simulink library "Renault_Library"  (Simulink 9.2)
settings: Solver=ode3  StartTime=0.0  StopTime=10.0  FixedStep=0.020  RelTol=1e-3  EnableMultiTasking=on
undefined symbols (not defined in the file or in its folder): ABC, AssystemProposal, b, chkval, CoeffA_C, CoeffB_C, CoeffMean_C, ConfTime_C, DiagDelay_C, DividePercent, FailureConfActive_C, FallingEdgeDuration_C, HighLevelThresholdVoltage_C, HighLevelVoltage_C, HighLevelVoltageTunable_C, InitValue_C, InputVoltRangeMax_C, InputVoltRangeMin_C, LowActiveContact_C, LowLevelThresholdVoltage_C, LowLevelVoltage_C, MaxOperatingVoltage_C, MinOperatingVoltage_C, mV2V_conversion, OUT1, OutOfRangeHigh_C, OutOfRangeLow_C, PinSupplySaturationMax, PinSupplySaturationMin, Res1Value_C, Res2Value_C, Res3Value_C, RisingEdgeDuration_C, RSA_CANSignalFailMonitoring, SampleTime_C, service14Table, service19Table, service22Table, service24Table, service28Table, service2ETable, service2FTable, service31Table, service87Table, SizeXStateDID2FTable, SizeXTable14, SizeXTable19, SizeXTable22, SizeXTable24, SizeXTable28, SizeXTable2E, SizeXTable2F, SizeXTable31, SizeXTable87, SizeYStateDID2FTable, SizeYTable14, SizeYTable19, SizeYTable22, SizeYTable24, SizeYTable28 ... (+22)
undefined symbols (not defined in the file): BatteryVoltage_ActivationThreshold_C, FrameStatus_AbsentValue_C, FrameStatus_ConfAbsentValue_C, MemThreshold_C, NAMEOFTHEMODEL_SAMPLE_TIME_CST, NbAbs1_C, NbConfAbs1_C, NbConfPres1_C, NbPres1_C, NOMDUSIGNAL_FaultConfTime_C, NOMDUSIGNAL_FaultRehabTime_C, NOMDUSIGNAL_switchvalue_C, NOMDUSIGNAL_unavailablevalue_C, PeriodFrame1_C, ResetTime_C, WakeUpTime_C
libraries: $bdroot x112, simulink x75
(part 8 of 32)

== system Renault_Library/Standard blocks/Logic & Bit Operations ==
  note: /!\ DO NOT USE THIS RED BLOCKS ANYMORE /!\
  note: Logic And Bit Operation
  Bit_Clear1 : simulink/Logic and Bit Operations/Bit Clear  SystemSampleTime=-1  iBit=0
      settings: SourceProductName=Simulink  SourceProductBaseCode=SL
  Bit_Set : simulink/Logic and Bit Operations/Bit Set  SystemSampleTime=-1  iBit=0
      settings: SourceProductName=Simulink  SourceProductBaseCode=SL
  BitwiseOperator : S-Function  FunctionName=sfix_bitop  Parameters=UseBitMask,NumInputPorts,BitMask,BitMaskRealWorld,logicop  SFunctionDeploymentMode=off  EnableBusSupport=off  mask(Bitwise Operator){logicop=AND, UseBitMask=on, NumInputPorts=1, BitMask=bin2dec('11011001'), BitMaskRealWorld=Stored Integer}
      mask prompts: logicop "Operator:"; UseBitMask "Use bit mask ..."; NumInputPorts "Number of input ports:"; BitMask "Bit Mask"; BitMaskRealWorld "Treat mask as:"
      mask help: Perform the specified bitwise operation on the inputs. The output data type should represent zero exactly.
      mask init:
      | str = '';
      | if UseBitMask
      |   [m,n] = size(BitMask);
      |   if ischar(BitMask)
      |     len = m;
      |     n = 1;
      |   else
      |     len = m * n;
      |   end
      |   internalBitMask = zeros(m,n);
      |   if isnumeric(BitMask)
      |     for i=1:len,
      |       internalBitMask(i) = BitMask(i);
      |     end
      |   elseif ischar(BitMask)
      |     for i=1:m,
      |       internalBitMask(i) = hex2dec(BitMask(i,:));
      |     end
      |   elseif iscell(BitMask)
      |     for i=1:len,
      |       if isnumeric( BitMask{i} )
      |         internalBitMask(i) = BitMask{i};
      |       elseif ischar( BitMask{i} )
      |         internalBitMask(i) = hex2dec(BitMask{i});
      |       else
      |         errordlg(['Data type is not supported at cell item ', num2str(i) ]);
      |       end
      |     end
      |   end
      |   BitMask = internalBitMask;
      |   for i=1:len
      |     if BitMask(i) > (2^52)-1
      |       error(DAStudio.message('Simulink:blocks:BitMaskWordLengthTooLarge')); 
      |     end
      |   end 
      |   if len == 1
      |      if BitMaskRealWorld == 1 || BitMask(1) < 0
      |        str=num2str(BitMask(1));
      |      else
      |        str=['0x',dec2hex(floor(BitMask(1)))];
      |      end
      |   else
      |      str='Masks';
      |   end
      | else
      |   BitMask = 0;
      |   str='';
      | end
      mask TypeOptions logicop: AND | OR | NAND | NOR | XOR | NOT
      mask Callback logicop: fixptdialog(gcbh)
      mask Callback UseBitMask: fixptdialog(gcbh)
      mask TypeOptions BitMaskRealWorld: Real World Value | Stored Integer
      mask Display: fprintf(['Bitwise\n',logicop,'\n',str])
  Bitwise_Operator : simulink/Logic and Bit Operations/Bitwise Operator  logicop=AND  UseBitMask=on  BitMask=bin2dec('11011001')  BitMaskRealWorld=Stored Integer
  Extract Bits : Subsystem  mask(Extract Bits){bitsToExtract=Upper half, numBits=8, bitIdxRange=[0 7], outScalingMode=Preserve fixed-point scaling}
      mask prompts: bitsToExtract "Bits to extract:"; numBits "Number of bits:"; bitIdxRange "Bit indices ([start end], 0-based relative to LSB):"; outScalingMode "Output scaling mode:"
      mask help: Output selected bits from each of the fixed-point input samples. Selecting "Upper half" or "Lower half" results in a positive number of bits in the output word length, according to the equation numOutputBits = ceil(numInputBits / 2).
      mask init:
      | str = fixpt_extractbits_mask('icon', numBits, bitIdxRange); 
      | fixpt_extractbits_mask_script;
      mask TypeOptions bitsToExtract: Upper half | Lower half | Range starting with most significant bit | Range ending with least significant bit | Range of bits
      mask Callback bitsToExtract: fixpt_extractbits_mask;
      mask TypeOptions outScalingMode: Preserve fixed-point scaling | Treat bit field as an integer
      mask Display: disp(str);
  Extract_Bits : simulink/Logic and Bit Operations/Extract Bits  SystemSampleTime=-1  bitsToExtract=Upper half  numBits=8  bitIdxRange=[0 7]  outScalingMode=Preserve fixed-point scaling
      settings: SourceProductName=Simulink
  LogicalOperator : Logic  OutDataTypeStr=boolean
  RelationalOperator : RelationalOperator  Operator=<=  OutDataTypeStr=boolean  RndMeth=Nearest  InputSameDT=on
  ShiftArithmetic : ArithShift  BitShiftNumber=8

== system Renault_Library/Standard blocks/Math Operations ==
  note: Math Operations
  Abs : Abs
  Add : Sum  OutDataTypeStr=Inherit: Inherit via back propagation  RndMeth=Zero  InputSameDT=on
      settings: AttributesFormatString=SAT = %<SaturateOnIntegerOverflow>
  Assignment : Assignment  IndexOptions=Index vector (dialog)  Indices=1  OutputSizes=1
  Divide : Product  Inputs=*/  RndMeth=Zero
  MinMax : MinMax  RndMeth=Zero  InputSameDT=on
  Product : Product  RndMeth=Zero
  Sign : Signum
  SignedSqrt : Sqrt  Operator=signedSqrt  SaturateOnIntegerOverflow=on
  Sqrt : Sqrt  SaturateOnIntegerOverflow=on
  Subtract : Sum  Inputs=+-  OutDataTypeStr=Inherit: Inherit via back propagation  RndMeth=Zero  InputSameDT=on
      settings: AttributesFormatString=SAT = %<SaturateOnIntegerOverflow>
  Sum : Sum  Inputs=|++  OutDataTypeStr=Inherit: Inherit via back propagation  RndMeth=Zero  InputSameDT=on
      settings: AttributesFormatString=SAT = %<SaturateOnIntegerOverflow>
  SumofElements : Sum  Inputs=+  OutDataTypeStr=Inherit: Inherit via back propagation  RndMeth=Zero  InputSameDT=on
      settings: AttributesFormatString=SAT = %<SaturateOnIntegerOverflow>

== system Renault_Library/Standard blocks/Model Wide Utilities ==
  note: /!\ DO NOT USE THIS RED BLOCKS ANYMORE /!\
  note: Model Wide Utilities
  DocBlock : Subsystem  mask(DocBlock){ECoderFlag=, DocumentType=Text}
      mask prompts: ECoderFlag "Simulink:blocks:docblockEmbeddedCoderFlagLabel"; DocumentType "Simulink:blocks:docblockDocTypeLabel"
      mask help: Use this block to save long descriptive text with the model. Double-clicking the block will open an editor.
      settings: DeleteFcn=docblock('close_document',gcb);  PreSaveFcn=docblock('save_document',gcb);  OpenFcn=docblock('edit_document',gcb);
      mask TypeOptions DocumentType: Text | RTF | HTML
      mask Display:
      | plot([.8 0 0 1 1 .8 .8 1],[1 1 0 0 .8 1 .8 .8]);
      | text(.5,.6,'DOC','horizontalalignment','center');
      | text(.95,.05,get_param(gcb,'DocumentType'),'verticalalignment','bottom','horizontalalignment','right');
  ModelInfo : Subsystem  LoadFcn=slcm LoadBlock;  InitFcn=slcm('UpdateAllCMBlocksInThisModelInit')  mask(CMBlock){InitialSaveTempField=, InitialBlockCM=None, BlockCM=none, Frame=on, SaveTempField=, DisplayStringWithTags=Model Info, MaskDisplayString=Model Info, HorizontalTextAlignment=Center, LeftAlignmentValue=0.5, SourceBlockDiagram=Renault_Library, TagMaxNumber=20, CMTag1=, CMTag2=, CMTag3=, CMTag4=, CMTag5=, CMTag6=, CMTag7=, CMTag8=, CMTag9=, CMTag10=, CMTag11=, CMTag12=, CMTag13=, CMTag14=, CMTag15=, CMTag16=, CMTag17=, CMTag18=, CMTag19=, CMTag20=}
      mask help: This block allows revision control information to be displayed within the model.
      settings: CopyFcn=slcm CopyBlock;  DeleteFcn=slcm Cancel;  PostSaveFcn=slcm('UpdateAllCMBlocksInThisModel')  NameChangeFcn=slcm Rename;  OpenFcn=slcm
      mask Display: text(str2num(LeftAlignmentValue), 0.5, MaskDisplayString, 'HorizontalAlignment', HorizontalTextAlignment)
  Model_Info : simulink/Model-Wide Utilities/Model Info  SystemSampleTime=-1  InitialBlockCM=none  BlockCM=none  Frame=on  DisplayStringWithTags=Model Info  HorizontalTextAlignment=Center  LeftAlignmentValue=0.5  SourceBlockDiagram=Renault_Library_11  TagMaxNumber=20

== system Renault_Library/Standard blocks/Ports & Subsystems ==
  note: Ports and Subsystems
  In : Inport
  Enable : EnablePort
      settings: AttributesFormatString=State : %<StatesWhenEnabling>
  Trigger : TriggerPort  VariantControl=(inherit)
      settings: AttributesFormatString=State : %<StatesWhenEnabling>
  AtomicSubsystem : Subsystem  TreatAsAtomicUnit=on  mask(Atomic Subsystem){}
      mask help: A subsystem block template containing an inport and outport block.
      settings: CopyFcn=set_param(gcbh,'LinkStatus','none'); set_param(gcbh,'Mask','off'); set_param(gcbh,'MaskType',''); set_param(gcbh,'MaskDescription',''); set_param(gcbh,'OpenFcn',''); set_param(gcbh,'CopyFcn','')  OpenFcn=open_system(gcbh,'force')
  CodeReuseSubsystem : Subsystem  TreatAsAtomicUnit=on  mask(Code Reuse Subsystem){}
      mask help: A subsystem block template containing an inport and outport block.
      settings: CopyFcn=set_param(gcbh,'LinkStatus','none'); set_param(gcbh,'Mask','off'); set_param(gcbh,'MaskType',''); set_param(gcbh,'MaskDescription',''); set_param(gcbh,'OpenFcn',''); set_param(gcbh,'CopyFcn','')  OpenFcn=open_system(gcbh,'force')  RTWFcnNameOpts=Use subsystem name  RTWFileNameOpts=Use function name
  EnabledSubsystem : Subsystem  TreatAsAtomicUnit=on  mask(Enabled Subsystem){}
      mask help: A subsystem block template containing an enable port, inport and outport block.
      settings: CopyFcn=set_param(gcbh,'LinkStatus','none'); set_param(gcbh,'Mask','off'); set_param(gcbh,'MaskType',''); set_param(gcbh,'MaskDescription',''); set_param(gcbh,'OpenFcn',''); set_param(gcbh,'CopyFcn','')  OpenFcn=open_system(gcbh,'force')
  EnabledandTriggeredSubsystem : Subsystem  TreatAsAtomicUnit=on  mask(Enabled And Triggered Subsystem){}
      mask help: A subsystem block template containing an enable port, trigger port, inport and outport block.
      settings: CopyFcn=set_param(gcbh,'LinkStatus','none'); set_param(gcbh,'Mask','off'); set_param(gcbh,'MaskType',''); set_param(gcbh,'MaskDescription',''); set_param(gcbh,'OpenFcn',''); set_param(gcbh,'CopyFcn','');  OpenFcn=open_system(gcbh,'force');
  ForIteratorSubsystem : Subsystem  TreatAsAtomicUnit=on  mask(For Iterator Subsystem){}
      mask help: A subsystem block template containing a for iterator, inport and outport block.
      settings: CopyFcn=set_param(gcbh,'LinkStatus','none'); set_param(gcbh,'Mask','off'); set_param(gcbh,'MaskType',''); set_param(gcbh,'MaskDescription',''); set_param(gcbh,'OpenFcn',''); set_param(gcbh,'CopyFcn','')  OpenFcn=open_system(gcbh,'force')
  FunctionCallSubsystem : Subsystem  TreatAsAtomicUnit=on  mask(Function-Call Subsystem){}
      mask help: A subsystem block template containing a function-call trigger port, inport and outport block.
      settings: CopyFcn=set_param(gcbh,'LinkStatus','none'); set_param(gcbh,'Mask','off'); set_param(gcbh,'MaskType',''); set_param(gcbh,'MaskDescription',''); set_param(gcbh,'OpenFcn',''); set_param(gcbh,'CopyFcn','');  OpenFcn=open_system(gcbh,'force');
  FunctionCall_Generator : simulink/Ports & Subsystems/Function-Call Generator  sample_time=1  numberOfIterations=1
  If : If
  IfActionSubsystem : Subsystem  TreatAsAtomicUnit=on  mask(If Action Subsystem){}
      mask help: A subsystem block template containing an action port, inport and outport block.
      settings: CopyFcn=set_param(gcbh,'LinkStatus','none'); set_param(gcbh,'Mask','off'); set_param(gcbh,'MaskType',''); set_param(gcbh,'MaskDescription',''); set_param(gcbh,'OpenFcn',''); set_param(gcbh,'CopyFcn','')  OpenFcn=open_system(gcbh,'force')
  Model : ModelReference  SimulationMode=Normal
  Model_Variants : ModelReference  SimulationMode=Normal  Variant=on
      settings: AttributesFormatString=%<ActiveVariant>
  Subsystem : Subsystem  mask(Virtual Subsystem){}
      mask help: A subsystem block template containing an inport and outport block.
      settings: CopyFcn=set_param(gcbh,'LinkStatus','none'); set_param(gcbh,'Mask','off'); set_param(gcbh,'MaskType',''); set_param(gcbh,'MaskDescription',''); set_param(gcbh,'OpenFcn',''); set_param(gcbh,'CopyFcn','')  OpenFcn=open_system(gcbh,'force')
  SwitchCase : SwitchCase
  SwitchCaseActionSubsystem : Subsystem  TreatAsAtomicUnit=on  mask(Switch Case Action Subsystem){}
      mask help: A subsystem block template containing an action port, inport and outport block.
      settings: CopyFcn=set_param(gcbh,'LinkStatus','none'); set_param(gcbh,'Mask','off'); set_param(gcbh,'MaskType',''); set_param(gcbh,'MaskDescription',''); set_param(gcbh,'OpenFcn',''); set_param(gcbh,'CopyFcn','')  OpenFcn=open_system(gcbh,'force')
  SwitchCaseActionSubsystem1 : Subsystem  TreatAsAtomicUnit=on  mask(Switch Case Action Subsystem){}
      mask help: A subsystem block template containing an action port, inport and outport block.
      settings: CopyFcn=set_param(gcbh,'LinkStatus','none'); set_param(gcbh,'Mask','off'); set_param(gcbh,'MaskType',''); set_param(gcbh,'MaskDescription',''); set_param(gcbh,'OpenFcn',''); set_param(gcbh,'CopyFcn','')  OpenFcn=open_system(gcbh,'force')
  TriggeredSubsystem : Subsystem  TreatAsAtomicUnit=on  mask(Triggered Subsystem){}
      mask help: A subsystem block template containing a trigger port, inport and outport block.
      settings: CopyFcn=set_param(gcbh,'LinkStatus','none'); set_param(gcbh,'Mask','off'); set_param(gcbh,'MaskType',''); set_param(gcbh,'MaskDescription',''); set_param(gcbh,'OpenFcn',''); set_param(gcbh,'CopyFcn','')  OpenFcn=open_system(gcbh,'force')
  Variant_Subsystem : Subsystem  Variant=on  mask(Variant Subsystem){}
      mask help: A Variant Subsystem template containing an inport and outport block
      settings: CopyFcn=set_param(gcbh,'LinkStatus','none'); set_param(gcbh,'Mask','off'); set_param(gcbh,'MaskType',''); set_param(gcbh,'MaskDescription',''); set_param(gcbh,'OpenFcn',''); set_param(gcbh,'CopyFcn','')
  WhileIteratorSubsystem : Subsystem  TreatAsAtomicUnit=on  mask(While Iterator Subsystem){}
      mask help: A subsystem block template containing a while iterator, inport and outport block.
      settings: CopyFcn=set_param(gcbh,'LinkStatus','none'); set_param(gcbh,'Mask','off'); set_param(gcbh,'MaskType',''); set_param(gcbh,'MaskDescription',''); set_param(gcbh,'OpenFcn',''); set_param(gcbh,'CopyFcn','')  OpenFcn=open_system(gcbh,'force')
  Out : Outport
  wires:
  SwitchCase/2 -> SwitchCaseActionSubsystem/Action
  SwitchCase/1 -> SwitchCaseActionSubsystem1/Action
  If/1 -> IfActionSubsystem/Action

== system Renault_Library/Standard blocks/Signal Attributes ==
  note: Signal Attibutes
  DataTypeConversion : DataTypeConversion  RndMeth=Zero

== system Renault_Library/Standard blocks/Signal Routing ==
  note: Signal Routing
  BusCreator2 : BusCreator  Inputs=2
  BusSelector2 : BusSelector  OutputSignals=signal1,signal2
  Demux2 : Demux  Outputs=2
  IndexVector : MultiPortSwitch  DataPortOrder=Zero-based contiguous  Inputs=1  zeroidx=on  RndMeth=Zero  InputSameDT=on
      settings: AttributesFormatString=PortOrder=%<DataPortOrder>
  Merge : Merge
  MultiportSwitch : MultiPortSwitch  DataPortOrder=Zero-based contiguous  zeroidx=on  RndMeth=Zero  InputSameDT=on
      settings: AttributesFormatString=PortOrder=%<DataPortOrder>
  Mux2 : Mux  Inputs=2
  Selector : Selector  InputPortWidth=3  IndexOptions=Index vector (dialog)  Indices=[1 3]  OutputSizes=1
  Switch : Switch  Criteria=u2 ~= 0  RndMeth=Zero  InputSameDT=on
      settings: AttributesFormatString=Criteria=%<Criteria>

== system Renault_Library/Standard blocks/Sinks ==
  note: Sink
  Terminator : Terminator
  Out : Outport

== system Renault_Library/Standard blocks/Sources ==
  note: Sources
  In : Inport
  Constant : Constant  Value=ABC
  Enumerated_Constant : simulink/Sources/Enumerated Constant  SystemSampleTime=-1  OutDataTypeStr=Enum: SlDemoSign  Value=SlDemoSign.Positive  SampleTime=-1
      settings: SourceProductName=Simulink

== system Renault_Library/Complex blocks/Discontinuities/RSA_DetectionSaturation ==
  note: 0 : pas saturation
      | 1: saturation min
      |  2: saturation max
      | 3: saturation avec min limite = min limite
  Threshold_Upper : Inport
      settings: AttributesFormatString=Type : %<OutDataTypeStr>
  In : Inport  Port=2
      settings: AttributesFormatString=Type : %<OutDataTypeStr>
  Threshold_Lower : Inport  Port=3
      settings: AttributesFormatString=Type : %<OutDataTypeStr>
  Constant : Constant  Value=SATMIN  OutDataTypeStr=Inherit: Inherit via back propagation  SampleTime=-1
  Constant1 : Constant  Value=SATMAX  OutDataTypeStr=Inherit: Inherit via back propagation  SampleTime=-1
  Constant2 : Constant  Value=SATPAS  OutDataTypeStr=uint8  SampleTime=-1
  Max : MinMax  Function=max  Inputs=2  OutDataTypeStr=Inherit: Inherit via back propagation  RndMeth=Zero  SaturateOnIntegerOverflow=on  InputSameDT=on
  Min : MinMax  Inputs=2  OutDataTypeStr=Inherit: Inherit via back propagation  RndMeth=Zero  SaturateOnIntegerOverflow=on  InputSameDT=on
  RelationalOperator : RelationalOperator  Operator===  OutDataTypeStr=boolean  RndMeth=Nearest  InputSameDT=on
  RelationalOperator1 : RelationalOperator  Operator===  OutDataTypeStr=boolean  RndMeth=Nearest  InputSameDT=on
  Sum : Sum  Inputs=2  OutDataTypeStr=Inherit: Inherit via back propagation  RndMeth=Zero  SaturateOnIntegerOverflow=on  InputSameDT=on
      settings: AttributesFormatString=SAT = %<SaturateOnIntegerOverflow>
  Switch : Switch  Criteria=u2 ~= 0  RndMeth=Zero  InputSameDT=on
      settings: AttributesFormatString=Criteria=%<Criteria>
  Switch1 : Switch  Criteria=u2 ~= 0  RndMeth=Zero  InputSameDT=on
      settings: AttributesFormatString=Criteria=%<Criteria>
  Out : Outport
      settings: AttributesFormatString=Type : %<OutDataTypeStr>\nInit :%<InitialOutput>
  sat : Outport  Port=2
      settings: AttributesFormatString=Type : %<OutDataTypeStr>\nInit :%<InitialOutput>
  wires:
  In/1 -> Max/1
  Threshold_Lower/1 -> RelationalOperator1/2, Max/2
  Max/1 -> Min/1
  Min/1 -> RelationalOperator1/1, RelationalOperator/1, Out/1
  RelationalOperator/1 -> Switch/2
  Sum/1 -> sat/1
  Switch/1 -> Sum/1
  Switch1/1 -> Sum/2
  RelationalOperator1/1 -> Switch1/2
  Constant/1 -> Switch1/1
  Constant1/1 -> Switch/1
  [Nsx_Zero] -> Switch1/3
  [Nsx_Zero] -> Switch/3
  Constant2/1 -> [Nsx_Zero]
  Threshold_Upper/1 -> [Threshold_Upper]
  [Threshold_Upper] -> Min/2
  [Threshold_Upper] -> RelationalOperator/2

== system Renault_Library/Complex blocks/Discontinuities/RSA_Hysteresis ==
  High_Threshold : Inport
      settings: AttributesFormatString=Type : %<OutDataTypeStr>
  In : Inport  Port=2
      settings: AttributesFormatString=Type : %<OutDataTypeStr>
  Low_Threshold : Inport  Port=3
      settings: AttributesFormatString=Type : %<OutDataTypeStr>
  DataTypeConversion : DataTypeConversion  RndMeth=Zero
  FixPt_Relational_Operator : RelationalOperator  OutDataTypeStr=boolean  ZeroCross=off  RndMeth=Nearest  InputSameDT=on
  FixPt_Relational_Operator1 : RelationalOperator  Operator=<=  OutDataTypeStr=boolean  ZeroCross=off  RndMeth=Nearest  InputSameDT=on
  Logical_Operator : Logic  Operator=OR  OutDataTypeStr=boolean
  Logical_Operator1 : Logic  Operator=NOT  OutDataTypeStr=boolean
  Logical_Operator2 : Logic  OutDataTypeStr=boolean
  Unit_Delay : UnitDelay  InitialCondition=HYSINI  SampleTime=-1
  Out : Outport
      settings: AttributesFormatString=Type : %<OutDataTypeStr>\nInit :%<InitialOutput>
  wires:
  DataTypeConversion/1 -> Out/1
  [In] -> FixPt_Relational_Operator/1
  In/1 -> [In]
  [In] -> FixPt_Relational_Operator1/1
  FixPt_Relational_Operator1/1 -> Logical_Operator1/1
  FixPt_Relational_Operator/1 -> Logical_Operator/2
  Low_Threshold/1 -> FixPt_Relational_Operator1/2
  High_Threshold/1 -> FixPt_Relational_Operator/2
  Unit_Delay/1 -> Logical_Operator/1
  Logical_Operator2/1 -> DataTypeConversion/1, Unit_Delay/1  "X3"
  Logical_Operator1/1 -> Logical_Operator2/2  "X2"
  Logical_Operator/1 -> Logical_Operator2/1  "X1"

== system Renault_Library/Complex blocks/Discontinuities/RSA_Hysteresis1 ==
  Input : Inport  Tag=AM,In,0,1,Name,C;CMP,Port,PortWidth,SampleTime;
      settings: AttributesFormatString=Type : %<OutDataTypeStr>
  Threshold1 : Inport  Tag=AM,In,0,1,Name,C;CMP,Port,PortWidth,SampleTime;  Port=2
      settings: AttributesFormatString=Type : %<OutDataTypeStr>
  Threshold2 : Inport  Tag=AM,In,0,1,Name,C;CMP,Port,PortWidth,SampleTime;  Port=3
      settings: AttributesFormatString=Type : %<OutDataTypeStr>
  Value1 : Inport  Tag=AM,In,0,1,Name,C;CMP,Port,PortWidth,SampleTime;  Port=4
      settings: AttributesFormatString=Type : %<OutDataTypeStr>
  Value2 : Inport  Tag=AM,In,0,1,Name,C;CMP,Port,PortWidth,SampleTime;  Port=5
      settings: AttributesFormatString=Type : %<OutDataTypeStr>
  DefaultValue : Inport  Tag=AM,In,0,1,Name,C;CMP,Port,PortWidth,SampleTime;  Port=6
      settings: AttributesFormatString=Type : %<OutDataTypeStr>
  Constant1 : Constant  Value=Th1_Strict  OutDataTypeStr=boolean  SampleTime=-1
  Constant2 : Constant  Value=Th2_Strict  OutDataTypeStr=boolean  SampleTime=-1
  DataTypeConversion : DataTypeConversion  RndMeth=Zero
  RSA_UnitDelayExternalIC : $bdroot/Complex blocks/Discrete/RSA_UnitDelayExternalIC  Tag=[LIB]
  RelationalOperator : RelationalOperator  Tag=AM,Std,2,1;CMP,Operator;  OutDataTypeStr=boolean  RndMeth=Nearest  InputSameDT=on
  RelationalOperator1 : RelationalOperator  Tag=AM,Std,2,1;CMP,Operator;  OutDataTypeStr=boolean  RndMeth=Nearest  InputSameDT=on
  RelationalOperator2 : RelationalOperator  Tag=AM,Std,2,1;CMP,Operator;  Operator=>  OutDataTypeStr=boolean  RndMeth=Nearest  InputSameDT=on
  RelationalOperator3 : RelationalOperator  Tag=AM,Std,2,1;CMP,Operator;  Operator=>  OutDataTypeStr=boolean  RndMeth=Nearest  InputSameDT=on
  Switch : Switch  Tag=GEN,Threshold;AM,Switch,2,1;CMP,Threshold;  Criteria=u2 ~= 0  RndMeth=Zero  SaturateOnIntegerOverflow=on  InputSameDT=on
      settings: AttributesFormatString=Criteria=%<Criteria>
  Switch1 : Switch  Tag=GEN,Threshold;AM,Switch,2,1;CMP,Threshold;  Criteria=u2 ~= 0  RndMeth=Zero  SaturateOnIntegerOverflow=on  InputSameDT=on
      settings: AttributesFormatString=Criteria=%<Criteria>
  Switch2 : Switch  Criteria=u2 ~= 0  RndMeth=Zero  InputSameDT=on
      settings: AttributesFormatString=Criteria=%<Criteria>
  Switch3 : Switch  Criteria=u2 ~= 0  RndMeth=Zero  InputSameDT=on
      settings: AttributesFormatString=Criteria=%<Criteria>
  Output : Outport  Tag=AM,Out,1,0,Name,NC;CMP,Port,OutputWhenDisabled,InitialOutput;
      settings: AttributesFormatString=Type : %<OutDataTypeStr>\nInit :%<InitialOutput>
  wires:
  RSA_UnitDelayExternalIC/1 -> Switch/3
  Switch/1 -> Switch1/3
  Switch1/1 -> RSA_UnitDelayExternalIC/1, DataTypeConversion/1
  Threshold2/1 -> [Threshold2]
  Threshold1/1 -> [Threshold1]
  [Value1] -> Switch1/1
  RelationalOperator1/1 -> Switch2/1
  Constant1/1 -> Switch2/2
  RelationalOperator2/1 -> Switch2/3
  RelationalOperator/1 -> Switch3/1
  Constant2/1 -> Switch3/2
  RelationalOperator3/1 -> Switch3/3
  Switch3/1 -> Switch/2
  Switch2/1 -> Switch1/2
  [Threshold1] -> RelationalOperator1/1
  [Threshold1] -> RelationalOperator2/1
  [Threshold2] -> RelationalOperator/2
  [Threshold2] -> RelationalOperator3/2
  Input/1 -> RelationalOperator1/2, RelationalOperator2/2, RelationalOperator/1, RelationalOperator3/1
  DefaultValue/1 -> [DefaultValue]
  [DefaultValue] -> RSA_UnitDelayExternalIC/2
  Value1/1 -> [Value1]
  Value2/1 -> [Value2]
  [Value2] -> Switch/1
  DataTypeConversion/1 -> Output/1

== system Renault_Library/Complex blocks/Discontinuities/RSA_Hysteresis1_v01_04 ==
  Input : Inport  Tag=AM,In,0,1,Name,C;CMP,Port,PortWidth,SampleTime;
  Threshold1 : Inport  Tag=AM,In,0,1,Name,C;CMP,Port,PortWidth,SampleTime;  Port=2
  Threshold2 : Inport  Tag=AM,In,0,1,Name,C;CMP,Port,PortWidth,SampleTime;  Port=3
  Value1 : Inport  Tag=AM,In,0,1,Name,C;CMP,Port,PortWidth,SampleTime;  Port=4
  Value2 : Inport  Tag=AM,In,0,1,Name,C;CMP,Port,PortWidth,SampleTime;  Port=5
  DefaultValue : Inport  Tag=AM,In,0,1,Name,C;CMP,Port,PortWidth,SampleTime;  Port=6
  Constant1 : Constant  Value=Th1_Strict  OutDataTypeStr=boolean  SampleTime=-1
  Constant2 : Constant  Value=Th2_Strict  OutDataTypeStr=boolean  SampleTime=-1
  RSA_UnitDelayExternalIC_v01_03 : $bdroot/Complex blocks/Discrete/RSA_UnitDelayExternalIC_v01_03  Tag=[LIB]  SystemSampleTime=-1
      settings: AttributesFormatString=%<MaskType> : Ver : 01.03
  RelationalOperator : RelationalOperator  Tag=AM,Std,2,1;CMP,Operator;  OutDataTypeStr=boolean  RndMeth=Nearest  InputSameDT=on
  RelationalOperator1 : RelationalOperator  Tag=AM,Std,2,1;CMP,Operator;  OutDataTypeStr=boolean  RndMeth=Nearest  InputSameDT=on
  RelationalOperator2 : RelationalOperator  Tag=AM,Std,2,1;CMP,Operator;  Operator=>  OutDataTypeStr=boolean  RndMeth=Nearest  InputSameDT=on
  RelationalOperator3 : RelationalOperator  Tag=AM,Std,2,1;CMP,Operator;  Operator=>  OutDataTypeStr=boolean  RndMeth=Nearest  InputSameDT=on
  Switch : Switch  Tag=GEN,Threshold;AM,Switch,2,1;CMP,Threshold;  Criteria=u2 ~= 0  RndMeth=Zero  SaturateOnIntegerOverflow=on  InputSameDT=on
      settings: AttributesFormatString=Criteria=%<Criteria>
  Switch1 : Switch  Tag=GEN,Threshold;AM,Switch,2,1;CMP,Threshold;  Criteria=u2 ~= 0  RndMeth=Zero  SaturateOnIntegerOverflow=on  InputSameDT=on
      settings: AttributesFormatString=Criteria=%<Criteria>
  Switch2 : Switch  Criteria=u2 ~= 0  RndMeth=Zero  InputSameDT=on
      settings: AttributesFormatString=Criteria=%<Criteria>
  Switch3 : Switch  Criteria=u2 ~= 0  RndMeth=Zero  InputSameDT=on
      settings: AttributesFormatString=Criteria=%<Criteria>
  Output : Outport  Tag=AM,Out,1,0,Name,NC;CMP,Port,OutputWhenDisabled,InitialOutput;
  wires:
  Switch1/1 -> Output/1, RSA_UnitDelayExternalIC_v01_03/1
  [Value2] -> Switch/1
  Value2/1 -> [Value2]
  Value1/1 -> [Value1]
  [DefaultValue] -> RSA_UnitDelayExternalIC_v01_03/2
  DefaultValue/1 -> [DefaultValue]
  Input/1 -> RelationalOperator3/1, RelationalOperator/1, RelationalOperator2/2, RelationalOperator1/2
  [Threshold2] -> RelationalOperator3/2
  [Threshold2] -> RelationalOperator/2
  [Threshold1] -> RelationalOperator2/1
  [Threshold1] -> RelationalOperator1/1
  Switch2/1 -> Switch1/2
  Switch3/1 -> Switch/2
  RelationalOperator3/1 -> Switch3/3
  Constant2/1 -> Switch3/2
  RelationalOperator/1 -> Switch3/1
  RelationalOperator2/1 -> Switch2/3
  Constant1/1 -> Switch2/2
  RelationalOperator1/1 -> Switch2/1
  [Value1] -> Switch1/1
  Threshold1/1 -> [Threshold1]
  Threshold2/1 -> [Threshold2]
  Switch/1 -> Switch1/3
  RSA_UnitDelayExternalIC_v01_03/1 -> Switch/3

== system Renault_Library/Complex blocks/Discontinuities/RSA_Hysteresis1_v01_05 ==
  Input : Inport  Tag=AM,In,0,1,Name,C;CMP,Port,PortWidth,SampleTime;
  Threshold1 : Inport  Tag=AM,In,0,1,Name,C;CMP,Port,PortWidth,SampleTime;  Port=2
  Threshold2 : Inport  Tag=AM,In,0,1,Name,C;CMP,Port,PortWidth,SampleTime;  Port=3
  Value1 : Inport  Tag=AM,In,0,1,Name,C;CMP,Port,PortWidth,SampleTime;  Port=4
  Value2 : Inport  Tag=AM,In,0,1,Name,C;CMP,Port,PortWidth,SampleTime;  Port=5
  DefaultValue : Inport  Tag=AM,In,0,1,Name,C;CMP,Port,PortWidth,SampleTime;  Port=6
  RO_Th1Strict : RelationalOperator  Tag=AM,Std,2,1;CMP,Operator;  OutDataTypeStr=boolean  RndMeth=Nearest  InputSameDT=on
  RO_Th2Strict : RelationalOperator  Tag=AM,Std,2,1;CMP,Operator;  Operator=>  OutDataTypeStr=boolean  RndMeth=Nearest  InputSameDT=on
  RSA_UnitDelayExternalIC_v01_03 : $bdroot/Complex blocks/Discrete/RSA_UnitDelayExternalIC_v01_03  Tag=[LIB]  SystemSampleTime=-1
      settings: AttributesFormatString=%<MaskType> : Ver : 01.03
  Switch : Switch  Tag=GEN,Threshold;AM,Switch,2,1;CMP,Threshold;  Criteria=u2 ~= 0  RndMeth=Zero  SaturateOnIntegerOverflow=on  InputSameDT=on
      settings: AttributesFormatString=Criteria=%<Criteria>
  Switch1 : Switch  Tag=GEN,Threshold;AM,Switch,2,1;CMP,Threshold;  Criteria=u2 ~= 0  RndMeth=Zero  SaturateOnIntegerOverflow=on  InputSameDT=on
      settings: AttributesFormatString=Criteria=%<Criteria>
  Output : Outport  Tag=AM,Out,1,0,Name,NC;CMP,Port,OutputWhenDisabled,InitialOutput;
  wires:
  Switch1/1 -> Output/1, RSA_UnitDelayExternalIC_v01_03/1
  [Value2] -> Switch/1
  Value2/1 -> [Value2]
  Value1/1 -> [Value1]
  [DefaultValue] -> RSA_UnitDelayExternalIC_v01_03/2
  DefaultValue/1 -> [DefaultValue]
  Input/1 -> RO_Th2Strict/1, RO_Th1Strict/2
  [Threshold2] -> RO_Th2Strict/2
  [Threshold1] -> RO_Th1Strict/1
  RO_Th1Strict/1 -> Switch1/2
  RO_Th2Strict/1 -> Switch/2
  [Value1] -> Switch1/1
  Threshold1/1 -> [Threshold1]
  Threshold2/1 -> [Threshold2]
  Switch/1 -> Switch1/3
  RSA_UnitDelayExternalIC_v01_03/1 -> Switch/3

== system Renault_Library/Complex blocks/Discontinuities/RSA_Hysteresis_V01_03 ==
  High_Threshold : Inport
      settings: AttributesFormatString=Type : %<OutDataTypeStr>
  In : Inport  Port=2
      settings: AttributesFormatString=Type : %<OutDataTypeStr>
  Low_Threshold : Inport  Port=3
      settings: AttributesFormatString=Type : %<OutDataTypeStr>
  DataTypeConversion : DataTypeConversion  RndMeth=Zero
  FixPt_Relational_Operator : RelationalOperator  OutDataTypeStr=boolean  ZeroCross=off  RndMeth=Nearest  InputSameDT=on
  FixPt_Relational_Operator1 : RelationalOperator  Operator=<=  OutDataTypeStr=boolean  ZeroCross=off  RndMeth=Nearest  InputSameDT=on
  Logical_Operator : Logic  Operator=OR  OutDataTypeStr=boolean
  Logical_Operator1 : Logic  Operator=NOT  OutDataTypeStr=boolean
  Logical_Operator2 : Logic  OutDataTypeStr=boolean
  Unit_Delay : UnitDelay  InitialCondition=HYSINI  SampleTime=-1
      settings: AttributesFormatString=IC = %<X0>
  Out : Outport
      settings: AttributesFormatString=Type : %<OutDataTypeStr>\nInit :%<InitialOutput>
  wires:
  DataTypeConversion/1 -> Out/1
  [In] -> FixPt_Relational_Operator/1
  In/1 -> [In]
  [In] -> FixPt_Relational_Operator1/1
  FixPt_Relational_Operator1/1 -> Logical_Operator1/1
  FixPt_Relational_Operator/1 -> Logical_Operator/2
  Low_Threshold/1 -> FixPt_Relational_Operator1/2
  High_Threshold/1 -> FixPt_Relational_Operator/2
  Unit_Delay/1 -> Logical_Operator/1
  Logical_Operator2/1 -> DataTypeConversion/1, Unit_Delay/1  "X3"
  Logical_Operator1/1 -> Logical_Operator2/2  "X2"
  Logical_Operator/1 -> Logical_Operator2/1  "X1"

== system Renault_Library/Complex blocks/Discontinuities/RSA_Hysteresis_WithConf_V01_00 ==
  Input : Inport  Tag=AM,In,0,1,Name,C;CMP,Port,PortWidth,SampleTime;
  Threshold1 : Inport  Tag=AM,In,0,1,Name,C;CMP,Port,PortWidth,SampleTime;  Port=2
  Threshold2 : Inport  Tag=AM,In,0,1,Name,C;CMP,Port,PortWidth,SampleTime;  Port=3
  Value1 : Inport  Tag=AM,In,0,1,Name,C;CMP,Port,PortWidth,SampleTime;  Port=4
  Value2 : Inport  Tag=AM,In,0,1,Name,C;CMP,Port,PortWidth,SampleTime;  Port=5
  DefaultValue : Inport  Tag=AM,In,0,1,Name,C;CMP,Port,PortWidth,SampleTime;  Port=6
  Timestep : Inport  Tag=AM,In,0,1,Name,C;CMP,Port,PortWidth,SampleTime;  Port=7
  ConfirmationTime : Inport  Tag=AM,In,0,1,Name,C;CMP,Port,PortWidth,SampleTime;  Port=8
  RO_Th1Strict : RelationalOperator  Tag=AM,Std,2,1;CMP,Operator;  OutDataTypeStr=boolean  RndMeth=Nearest  InputSameDT=on
  RO_Th2Strict : RelationalOperator  Tag=AM,Std,2,1;CMP,Operator;  Operator=>  OutDataTypeStr=boolean  RndMeth=Nearest  InputSameDT=on
  RSA_UnitDelayExternalIC_v01_03 : $bdroot/Complex blocks/Discrete/RSA_UnitDelayExternalIC_v01_03  Tag=[LIB]  SystemSampleTime=-1
      settings: AttributesFormatString=%<MaskType> : Ver : 01.03
  Switch : Switch  Tag=GEN,Threshold;AM,Switch,2,1;CMP,Threshold;  Criteria=u2 ~= 0  RndMeth=Zero  SaturateOnIntegerOverflow=on  InputSameDT=on
      settings: AttributesFormatString=Criteria=%<Criteria>
  Switch1 : Switch  Tag=GEN,Threshold;AM,Switch,2,1;CMP,Threshold;  Criteria=u2 ~= 0  RndMeth=Zero  SaturateOnIntegerOverflow=on  InputSameDT=on
      settings: AttributesFormatString=Criteria=%<Criteria>
  TurnOnDelay1 : Subsystem
  TurnOnDelay2 : Subsystem
  Output : Outport  Tag=AM,Out,1,0,Name,NC;CMP,Port,OutputWhenDisabled,InitialOutput;
  wires:
  Switch1/1 -> Output/1, RSA_UnitDelayExternalIC_v01_03/1
  [Value2] -> Switch/1
  Value2/1 -> [Value2]
  Value1/1 -> [Value1]
  [DefaultValue] -> RSA_UnitDelayExternalIC_v01_03/2
  DefaultValue/1 -> [DefaultValue]
  Input/1 -> RO_Th2Strict/1, RO_Th1Strict/2
  [Threshold2] -> RO_Th2Strict/2
  [Threshold1] -> RO_Th1Strict/1
  RO_Th1Strict/1 -> TurnOnDelay1/2(Signal)
  [Value1] -> Switch1/1
  Threshold1/1 -> [Threshold1]
  Threshold2/1 -> [Threshold2]
  Switch/1 -> Switch1/3
  RSA_UnitDelayExternalIC_v01_03/1 -> Switch/3
  TurnOnDelay1/1(Out) -> Switch1/2
  ConfirmationTime/1 -> [ConfirmationTime]
  Timestep/1 -> [Timestep]
  [Timestep] -> TurnOnDelay1/3(Timestep1)
  [ConfirmationTime] -> TurnOnDelay1/1(Delaytime)
  [Timestep] -> TurnOnDelay2/3(Timestep1)
  [ConfirmationTime] -> TurnOnDelay2/1(Delaytime)
  RO_Th2Strict/1 -> TurnOnDelay2/2(Signal)
  TurnOnDelay2/1(Out) -> Switch/2

== system Renault_Library/Complex blocks/Discontinuities/RSA_MaxSaturation ==
  u : Inport
      settings: AttributesFormatString=Type : %<OutDataTypeStr>
  Maximum : Constant  Value=Max  OutDataTypeStr=Inherit: Inherit via back propagation  SampleTime=-1  LockScale=on
  MinMax : MinMax  Inputs=2  OutDataTypeStr=Inherit: Inherit via back propagation  RndMeth=Zero  SaturateOnIntegerOverflow=on  InputSameDT=on
  output : Outport
      settings: AttributesFormatString=Type : %<OutDataTypeStr>\nInit :%<InitialOutput>
  wires:
  Maximum/1 -> MinMax/2
  u/1 -> MinMax/1
  MinMax/1 -> output/1

== system Renault_Library/Complex blocks/Discontinuities/RSA_MinSaturation ==
  u : Inport
      settings: AttributesFormatString=Type : %<OutDataTypeStr>
  MinMax : MinMax  Function=max  Inputs=2  OutDataTypeStr=Inherit: Inherit via back propagation  RndMeth=Zero  SaturateOnIntegerOverflow=on  InputSameDT=on
  Minimum : Constant  Value=Min  OutDataTypeStr=Inherit: Inherit via back propagation  SampleTime=-1
  Output : Outport
      settings: AttributesFormatString=Type : %<OutDataTypeStr>\nInit :%<InitialOutput>
  wires:
  Minimum/1 -> MinMax/2
  u/1 -> MinMax/1
  MinMax/1 -> Output/1

== system Renault_Library/Complex blocks/Discrete/RSA_TappedDelay ==
  In1 : Inport  PortDimensions=1
      settings: AttributesFormatString=Type : %<OutDataTypeStr>
  Add : Sum  OutDataTypeStr=Inherit: Inherit via back propagation  RndMeth=Zero  InputSameDT=on
      settings: AttributesFormatString=SAT = %<SaturateOnIntegerOverflow>
  Add1 : Sum  OutDataTypeStr=Inherit: Inherit via back propagation  RndMeth=Zero  InputSameDT=on
      settings: AttributesFormatString=SAT = %<SaturateOnIntegerOverflow>
  Add2 : Sum  Inputs=-+  OutDataTypeStr=Inherit: Inherit via back propagation  RndMeth=Zero  InputSameDT=on
      settings: AttributesFormatString=SAT = %<SaturateOnIntegerOverflow>
  Add3 : Sum  OutDataTypeStr=Inherit: Inherit via back propagation  RndMeth=Zero  InputSameDT=on
      settings: AttributesFormatString=SAT = %<SaturateOnIntegerOverflow>
  Constant : Constant  Value=A_NoOfDelay  OutDataTypeStr=Inherit: Inherit via back propagation  SampleTime=-1
  Constant14 : Constant  Value=Order  OutDataTypeStr=boolean  SampleTime=-1
  Constant16 : Constant  Value=InitialValue  OutDataTypeStr=Inherit: Inherit via back propagation  SampleTime=-1
  Constant2 : Constant  Value=TAPDINI  OutDataTypeStr=Inherit: Inherit via back propagation  SampleTime=-1
  Constant4 : Constant  Value=INIADD  OutDataTypeStr=Inherit: Inherit via back propagation  SampleTime=-1
  Constant9 : Constant  Value=INIVECSEL  OutDataTypeStr=uint32  SampleTime=-1
  Data_Type_Conversion : DataTypeConversion  RndMeth=Zero
  Logical_Operator : Logic  OutDataTypeStr=boolean
  MultiportSwitch : MultiPortSwitch  Inputs=2  RndMeth=Zero  InputSameDT=on
      settings: AttributesFormatString=PortOrder=%<DataPortOrder>
  Mux : Mux  Inputs=2
  Mux1 : Mux  Inputs=2
  RSA_CompareToZero : $bdroot/Complex blocks/Logic & Bit Operations/RSA_CompareToZero  Tag=[LIB]  SystemSampleTime=-1  relop===  LogicOutDataTypeMode=boolean
      settings: AttributesFormatString=%<MaskType> : Ver : 01.03\nOperator: %<relop>\nOutput data type mode: %<LogicOutDataTypeMode>\nEnable zero-crossing detection %<ZeroCross>
  RSA_CompareToZero1 : $bdroot/Complex blocks/Logic & Bit Operations/RSA_CompareToZero  Tag=[LIB]  SystemSampleTime=-1  relop===  LogicOutDataTypeMode=boolean
      settings: AttributesFormatString=%<MaskType> : Ver : 01.03\nOperator: %<relop>\nOutput data type mode: %<LogicOutDataTypeMode>\nEnable zero-crossing detection %<ZeroCross>
  RSA_CompareToZero2 : $bdroot/Complex blocks/Logic & Bit Operations/RSA_CompareToZero  Tag=[LIB]  SystemSampleTime=-1  relop===  LogicOutDataTypeMode=boolean
      settings: AttributesFormatString=%<MaskType> : Ver : 01.03\nOperator: %<relop>\nOutput data type mode: %<LogicOutDataTypeMode>\nEnable zero-crossing detection %<ZeroCross>
  RelationalOperator : RelationalOperator  Operator===  OutDataTypeStr=boolean  RndMeth=Nearest  InputSameDT=on
  Selector : Selector  IndexOptions=Index vector (dialog)  Indices=Selector  OutputSizes=1
  Selector1 : Selector  IndexOptions=Index vector (dialog)  Indices=Order_Selec_Inv  OutputSizes=1
  Selector2 : Selector  IndexOptions=Index vector (dialog)  Indices=Append  OutputSizes=1
  Selector3 : Selector  IndexOptions=Index vector (dialog)  Indices=Order_Selec_Req  OutputSizes=1
  Switch{1..4} (4 alike) : Switch  Criteria=u2 ~= 0  RndMeth=Zero  InputSameDT=on
      settings: AttributesFormatString=Criteria=%<Criteria>
  Unit_Delay{,1..4} (5 alike) : UnitDelay  InitialCondition=TAPDINI  SampleTime=-1
  Out1 : Outport
      settings: AttributesFormatString=Type : %<OutDataTypeStr>\nInit :%<InitialOutput>
  wires:
  Constant4/1 -> Add2/1, [Nsx_One], Add1/1
  Selector2/1 -> Selector3/1, Selector1/1
  [DelayedVector] -> RSA_CompareToZero1/1, Add3/1
  [DelayedVector] -> Unit_Delay3/1
  Mux/1 -> [DelayedVector]
  Switch3/1 -> Out1/1
  Selector3/1 -> Switch3/3
  Selector1/1 -> Switch3/1
  [In1] -> Mux1/1
  [In1] -> Unit_Delay4/1
  In1/1 -> [In1]
  Mux1/1 -> Selector2/1
  Add3/1 -> Mux1/2
  Constant/1 -> RelationalOperator/2
  Unit_Delay2/1 -> [InitialVector]
  [InitialCheck] -> Switch2/2
  Constant14/1 -> Switch3/2
  Unit_Delay4/1 -> Switch2/3
  Switch2/1 -> Mux/1
  Selector/1 -> Mux/2
  RSA_CompareToZero/1 -> [InitialCheck], Switch1/2
  Data_Type_Conversion/1 -> Add/1
  RelationalOperator/1 -> Data_Type_Conversion/1
  Add/1 -> [InitCon], Unit_Delay/1, Unit_Delay2/1
  Unit_Delay/1 -> Add/2
  Add2/1 -> RSA_CompareToZero/1, RelationalOperator/1
  Unit_Delay1/1 -> Add1/2
  Add1/1 -> Add2/2, Unit_Delay1/1
  RSA_CompareToZero1/1 -> Logical_Operator/1
  RSA_CompareToZero2/1 -> Logical_Operator/2
  [InitCon] -> RSA_CompareToZero2/1
  Constant2/1 -> Switch2/1, [Nsx_zero]
  [InitialVector] -> MultiportSwitch/2
  MultiportSwitch/1 -> Selector/1
  Unit_Delay3/1 -> MultiportSwitch/3
  Switch1/1 -> MultiportSwitch/1
  Constant9/1 -> Switch1/3
  [Nsx_One] -> Switch1/1
  Switch4/1 -> Add3/2
  Constant16/1 -> Switch4/1
  Logical_Operator/1 -> Switch4/2
  [Nsx_zero] -> Switch4/3

== system Renault_Library/Complex blocks/Discrete/RSA_TappedDelay_V01_03 ==
  In1 : Inport  PortDimensions=1
      settings: AttributesFormatString=Type : %<OutDataTypeStr>
  Add1 : Sum  OutDataTypeStr=Inherit: Inherit via back propagation  RndMeth=Zero  SaturateOnIntegerOverflow=on  InputSameDT=on
      settings: AttributesFormatString=SAT = %<SaturateOnIntegerOverflow>
  Add2 : Sum  Inputs=-+  OutDataTypeStr=Inherit: Inherit via back propagation  RndMeth=Zero  InputSameDT=on
      settings: AttributesFormatString=SAT = %<SaturateOnIntegerOverflow>
  Constant : Constant  Value=A_NoOfDelay  OutDataTypeStr=Inherit: Inherit via back propagation  SampleTime=-1
  Constant16 : Constant  Value=InitialValue  OutDataTypeStr=Inherit: Inherit via back propagation  SampleTime=-1
  Constant4 : Constant  Value=INIADD  OutDataTypeStr=uint32  SampleTime=-1
  Logical_Operator1 : Logic  Operator=OR  OutDataTypeStr=boolean
  Logical_Operator2 : Logic  Operator=NOT  OutDataTypeStr=boolean
  Mux : Mux  Inputs=2
  Mux1 : Mux  Inputs=2
  RelationalOperator : RelationalOperator  Operator===  OutDataTypeStr=boolean  RndMeth=Nearest  InputSameDT=on
  Selector : Selector  IndexOptions=Index vector (dialog)  Indices=Selector  OutputSizes=1
  Selector1 : Selector  IndexOptions=Index vector (dialog)  Indices=Order_Selec_Inv  OutputSizes=1
  Selector2 : Selector  IndexOptions=Index vector (dialog)  Indices=Append  OutputSizes=1
  Selector3 : Selector  IndexOptions=Index vector (dialog)  Indices=Order_Selec_Req  OutputSizes=1
  Switch4 : Switch  Criteria=u2 ~= 0  RndMeth=Zero  InputSameDT=on
      settings: AttributesFormatString=Criteria=%<Criteria>
  Terminator : Terminator
  Terminator1 : Terminator
  Unit_Delay{,1,3..4} (4 alike) : UnitDelay  InitialCondition=TAPDINI  SampleTime=-1
      settings: AttributesFormatString=IC = %<X0>
  Out1 : Outport
      settings: AttributesFormatString=Type : %<OutDataTypeStr>\nInit :%<InitialOutput>
  wires:
  Switch4/1 -> Mux1/2
  Logical_Operator2/1 -> Switch4/2
  [InitCon] -> Logical_Operator2/1
  Unit_Delay3/1 -> Selector/1
  [In1] -> Mux1/1
  [oldest_order] -> Terminator1/1
  [newest_order] -> Terminator/1
  Add2/1 -> RelationalOperator/1
  [DelayedVector] -> Switch4/3
  Constant16/1 -> Switch4/1
  Add1/1 -> Unit_Delay1/1, Add2/2
  Unit_Delay1/1 -> Add1/2
  Unit_Delay/1 -> Logical_Operator1/2
  Logical_Operator1/1 -> [InitCon], Unit_Delay/1
  RelationalOperator/1 -> Logical_Operator1/1
  Selector/1 -> Mux/2
  Unit_Delay4/1 -> Mux/1
  Constant/1 -> RelationalOperator/2
  Mux1/1 -> Selector2/1
  In1/1 -> [In1]
  [In1] -> Unit_Delay4/1
  Selector1/1 -> [oldest_order]
  Selector3/1 -> [newest_order]
  [newest_order] -> Out1/1
  Mux/1 -> [DelayedVector]
  [DelayedVector] -> Unit_Delay3/1
  Selector2/1 -> Selector1/1, Selector3/1
  Constant4/1 -> Add1/1, Add2/1

== system Renault_Library/Complex blocks/Discrete/RSA_UnitDelayExternalIC ==
  u : Inport
      settings: AttributesFormatString=Type : %<OutDataTypeStr>
  IC : Inport  Port=2
      settings: AttributesFormatString=Type : %<OutDataTypeStr>
  FixPtUnitDelay2 : UnitDelay  DisableCoverage=on  InitialCondition=true  SampleTime=-1
  FixPt_Constant : Constant  DisableCoverage=on  Value=false  OutDataTypeStr=boolean  SampleTime=-1
  FixPt_Unit_Delay1 : UnitDelay  DisableCoverage=on  InitialCondition=UDEIC_zero  SampleTime=-1
  Init : Switch  Criteria=u2 ~= 0  RndMeth=Zero  ZeroCross=off  InputSameDT=on
      settings: AttributesFormatString=Criteria=%<Criteria>
  RSA_DataTypeDuplicate_v01_03 : $bdroot/Complex blocks/Signal Routing/RSA_DataTypeDuplicate_v01_03  Tag=[LIB]  NoIn=3
      settings: AttributesFormatString=%<MaskType> : Ver : 01.03\nNo Of Inputs %<NoIn>
  y : Outport
      settings: AttributesFormatString=Type : %<OutDataTypeStr>\nInit :%<InitialOutput>
  wires:
  [IC] -> RSA_DataTypeDuplicate_v01_03/3
  FixPt_Unit_Delay1/1 -> Init/3
  Init/1 -> y/1, RSA_DataTypeDuplicate_v01_03/1
  FixPtUnitDelay2/1 -> Init/2
  FixPt_Constant/1 -> FixPtUnitDelay2/1
  u/1 -> FixPt_Unit_Delay1/1, RSA_DataTypeDuplicate_v01_03/2
  IC/1 -> [IC], Init/1

== system Renault_Library/Complex blocks/Discrete/RSA_UnitDelayExternalIC_v01_03 ==
  u : Inport
  IC : Inport  Port=2
  FixPtUnitDelay2 : UnitDelay  DisableCoverage=on  InitialCondition=false  SampleTime=-1
      settings: AttributesFormatString=IC = %<X0>
  FixPt_Constant : Constant  DisableCoverage=on  Value=true  OutDataTypeStr=boolean  SampleTime=-1
  FixPt_Unit_Delay1 : UnitDelay  DisableCoverage=on  InitialCondition=UDEIC_zero  SampleTime=-1
      settings: AttributesFormatString=IC = %<X0>
  Init : Switch  Criteria=u2 ~= 0  RndMeth=Zero  ZeroCross=off  InputSameDT=on
      settings: AttributesFormatString=Criteria=%<Criteria>
  y : Outport
  wires:
  u/1 -> FixPt_Unit_Delay1/1
  FixPt_Constant/1 -> FixPtUnitDelay2/1
  FixPtUnitDelay2/1 -> Init/2
  Init/1 -> y/1
  FixPt_Unit_Delay1/1 -> Init/1
  IC/1 -> Init/3

== system Renault_Library/Complex blocks/Flip-Flop/RSA_SRFlipFlopSetPrioritary ==
  S : Inport
      settings: AttributesFormatString=Type : %<OutDataTypeStr>
  R : Inport  Port=2
      settings: AttributesFormatString=Type : %<OutDataTypeStr>
  Constant : Constant  Value=true  OutDataTypeStr=boolean  SampleTime=-1
  Constant1 : Constant  Value=false  OutDataTypeStr=Inherit: Inherit via back propagation  SampleTime=-1
  DataTypeConversion : DataTypeConversion  RndMeth=Zero
  Switch : Switch  Criteria=u2 ~= 0  RndMeth=Zero  InputSameDT=on
      settings: AttributesFormatString=Criteria=%<Criteria>
  Switch1 : Switch  Criteria=u2 ~= 0  RndMeth=Zero  InputSameDT=on
      settings: AttributesFormatString=Criteria=%<Criteria>
  Unit_Delay : UnitDelay  InitialCondition=init  SampleTime=-1
  Q : Outport
      settings: AttributesFormatString=Type : %<OutDataTypeStr>\nInit :%<InitialOutput>
  wires:
  DataTypeConversion/1 -> Q/1
  [prev_val] -> Switch1/3
  Unit_Delay/1 -> [prev_val]
  Switch/1 -> DataTypeConversion/1, Unit_Delay/1  "X2"
  Constant1/1 -> Switch1/1
  Switch1/1 -> Switch/3  "X1"
  Constant/1 -> Switch/1
  R/1 -> Switch1/2
  S/1 -> Switch/2

== system Renault_Library/Complex blocks/Flip-Flop/RSA_SRFlipFlopSetPrioritary_v01_03 ==
  S : Inport
  R : Inport  Port=2
  Constant : Constant  Value=true  OutDataTypeStr=boolean  SampleTime=-1
  Constant1 : Constant  Value=false  OutDataTypeStr=Inherit: Inherit via back propagation  SampleTime=-1
  Switch : Switch  Criteria=u2 ~= 0  RndMeth=Zero  InputSameDT=on
      settings: AttributesFormatString=Criteria=%<Criteria>
  Switch1 : Switch  Criteria=u2 ~= 0  RndMeth=Zero  InputSameDT=on
      settings: AttributesFormatString=Criteria=%<Criteria>
  Unit_Delay : UnitDelay  InitialCondition=init  SampleTime=-1
      settings: AttributesFormatString=IC = %<X0>
  Q : Outport
  wires:
  Switch/1 -> Unit_Delay/1, Q/1
  Unit_Delay/1 -> Switch1/3
  S/1 -> Switch/2
  R/1 -> Switch1/2
  Constant/1 -> Switch/1
  Switch1/1 -> Switch/3
  Constant1/1 -> Switch1/1

== system Renault_Library/Complex blocks/Flip-Flop/RSA_SRFlipFlop_v01_02 ==
  Set : Inport
  ReSet : Inport  Port=2
  LogicalOperator : Logic  Operator=OR  OutDataTypeStr=boolean
  LogicalOperator1 : Logic  Operator=NOT  OutDataTypeStr=boolean
  LogicalOperator2 : Logic  OutDataTypeStr=boolean
  UnitDelay : UnitDelay  InitialCondition=IC  SampleTime=-1
      settings: AttributesFormatString=IC = %<X0>
  Out : Outport
  wires:
  UnitDelay/1 -> LogicalOperator/1
  ReSet/1 -> LogicalOperator1/1
  Set/1 -> LogicalOperator/2
  LogicalOperator2/1 -> Out/1, UnitDelay/1
  LogicalOperator1/1 -> LogicalOperator2/2
  LogicalOperator/1 -> LogicalOperator2/1

== system Renault_Library/Complex blocks/Flip-Flop/RSA_SRFlipFlop_v01_03 ==
  Set : Inport
  ReSet : Inport  Port=2
  Constant : Constant  Value=Indeterminate_Condition  OutDataTypeStr=Inherit: Inherit via back propagation
  LogicalOperator : Logic  Operator=OR  OutDataTypeStr=boolean
  LogicalOperator1 : Logic  Operator=NOT  OutDataTypeStr=boolean
  LogicalOperator2 : Logic  OutDataTypeStr=boolean
  LogicalOperator6 : Logic  OutDataTypeStr=boolean
  Switch3 : Switch  Criteria=u2 ~= 0  RndMeth=Zero  InputSameDT=on
      settings: AttributesFormatString=Criteria=%<Criteria>
  UnitDelay : UnitDelay  InitialCondition=IC  SampleTime=-1
      settings: AttributesFormatString=IC = %<X0>
  Out : Outport
  wires:
  [Reset] -> LogicalOperator1/1
  [Set] -> LogicalOperator/2
  Switch3/1 -> Out/1
  Constant/1 -> Switch3/1
  LogicalOperator6/1 -> Switch3/2
  LogicalOperator/1 -> LogicalOperator2/1
  LogicalOperator1/1 -> LogicalOperator2/2
  LogicalOperator2/1 -> Switch3/3, UnitDelay/1
  UnitDelay/1 -> LogicalOperator/1
  [Reset] -> LogicalOperator6/2
  [Set] -> LogicalOperator6/1
  ReSet/1 -> [Reset]
  Set/1 -> [Set]

== system Renault_Library/Complex blocks/Logic & Bit Operations/RSA_CompareToConstant_V01_04 ==
  u : Inport
      settings: AttributesFormatString=Type : %<OutDataTypeStr>
  Compare : RelationalOperator  Operator===  OutDataTypeStr=boolean  ZeroCross=off  RndMeth=Nearest  InputSameDT=on
  Constant : Constant  DisableCoverage=on  Value=Const  OutDataTypeStr=Inherit: Inherit via back propagation  SampleTime=-1
  y : Outport
      settings: AttributesFormatString=Type : %<OutDataTypeStr>\nInit :%<InitialOutput>
  wires:
  Compare/1 -> y/1
  Constant/1 -> Compare/2
  u/1 -> Compare/1
